simulink model "Demo_TrafficFlow_cs"  (Simulink 7.6)
settings: Solver=ode5  SolverMode=SingleTasking  StartTime=0.0  StopTime=60  FixedStep=1/20  RelTol=1e-3
PreLoadFcn:
  | check_NUTS_defined
  | try
  |     evalin('base', 'NUTS_defined;');
  | catch
  |     warningMsg = sprintf('PreScan Warning: \nUsing this model requires that MATLAB is configured for PreScan. The model may not display correctly.\n\n ==> Please open MATLAB with the dedicated buttons provided with PreScan');
  |     disp(warningMsg)
  |     warndlg(warningMsg,'WindowStyle','Modal');
  | end
  | 
  | model_closed = 0;
  | assignin('base',['model_closed_' bdroot],false);
InitFcn:
  | PreScanExperimentInitFcn(bdroot)
StopFcn:
  | PreScanExperimentStopFcn(bdroot)
undefined symbols (not defined in the file or in its folder): Advised_Speed_L1, Advised_Speed_L2, Advised_Speed_L3, DiscIntNeverNeededParam, spare_10, spare_5, spare_6, spare_7, spare_8, spare_9, State_L2, Throughput_L1, Throughput_L2, Throughput_L3, Velocity_L1, Velocity_L2, Velocity_L3
libraries: simulink x58, PreScanSimulinkLibrary x40, simulink_extras x32, PreScanUsefulBlocks x22, simulink_need_slupdate x3
(part 7 of 20)

== system Demo_TrafficFlow_cs/VISUMatrixSign_HighwayOverhang_2 ==
  MatrixSigns : Inport
  SubMuxVisuMatrixSign : Demux  Outputs=12
  visu_MatrixSign_1 : Subsystem  mask(ToSensorWorld){PortName=MatrixSign_1, PortIndex=690, PortWidth=1, TimeStep=1/20}
      mask prompts: PortName "Port Name [-]"; PortIndex "Index [-]"; PortWidth "Port Dimensions [-]"; TimeStep "Upload Period [s]"
      mask help: Sends signal(s) from Simulink to the Sensor World
      mask Help:
      | Sends signal(s) from Simulink to the Sensor World.
      | 
      | - Port Name [-]: specifies the name of the output terminal of the Simulink block.
      | - Index [-]: specifies the start position in the output vector sent to the Sensor World
      | - Port dimensions [-]: specifies the width (number of scalars) of the output port
      | - Upload period [s]: specifies the upload period (in seconds) of the port.
      mask Display: port_label('input', 1, PortName)
  visu_MatrixSign_10 : Subsystem  mask(ToSensorWorld){PortName=MatrixSign_4, PortIndex=699, PortWidth=1, TimeStep=1/20}
      mask prompts: PortName "Port Name [-]"; PortIndex "Index [-]"; PortWidth "Port Dimensions [-]"; TimeStep "Upload Period [s]"
      mask help: Sends signal(s) from Simulink to the Sensor World
      mask Help:
      | Sends signal(s) from Simulink to the Sensor World.
      | 
      | - Port Name [-]: specifies the name of the output terminal of the Simulink block.
      | - Index [-]: specifies the start position in the output vector sent to the Sensor World
      | - Port dimensions [-]: specifies the width (number of scalars) of the output port
      | - Upload period [s]: specifies the upload period (in seconds) of the port.
      mask Display: port_label('input', 1, PortName)
  visu_MatrixSign_11 : Subsystem  mask(ToSensorWorld){PortName=MatrixSign_5, PortIndex=700, PortWidth=1, TimeStep=1/20}
      mask prompts: PortName "Port Name [-]"; PortIndex "Index [-]"; PortWidth "Port Dimensions [-]"; TimeStep "Upload Period [s]"
      mask help: Sends signal(s) from Simulink to the Sensor World
      mask Help:
      | Sends signal(s) from Simulink to the Sensor World.
      | 
      | - Port Name [-]: specifies the name of the output terminal of the Simulink block.
      | - Index [-]: specifies the start position in the output vector sent to the Sensor World
      | - Port dimensions [-]: specifies the width (number of scalars) of the output port
      | - Upload period [s]: specifies the upload period (in seconds) of the port.
      mask Display: port_label('input', 1, PortName)
  visu_MatrixSign_12 : Subsystem  mask(ToSensorWorld){PortName=MatrixSign_6, PortIndex=701, PortWidth=1, TimeStep=1/20}
      mask prompts: PortName "Port Name [-]"; PortIndex "Index [-]"; PortWidth "Port Dimensions [-]"; TimeStep "Upload Period [s]"
      mask help: Sends signal(s) from Simulink to the Sensor World
      mask Help:
      | Sends signal(s) from Simulink to the Sensor World.
      | 
      | - Port Name [-]: specifies the name of the output terminal of the Simulink block.
      | - Index [-]: specifies the start position in the output vector sent to the Sensor World
      | - Port dimensions [-]: specifies the width (number of scalars) of the output port
      | - Upload period [s]: specifies the upload period (in seconds) of the port.
      mask Display: port_label('input', 1, PortName)
  visu_MatrixSign_2 : Subsystem  mask(ToSensorWorld){PortName=MatrixSign_2, PortIndex=691, PortWidth=1, TimeStep=1/20}
      mask prompts: PortName "Port Name [-]"; PortIndex "Index [-]"; PortWidth "Port Dimensions [-]"; TimeStep "Upload Period [s]"
      mask help: Sends signal(s) from Simulink to the Sensor World
      mask Help:
      | Sends signal(s) from Simulink to the Sensor World.
      | 
      | - Port Name [-]: specifies the name of the output terminal of the Simulink block.
      | - Index [-]: specifies the start position in the output vector sent to the Sensor World
      | - Port dimensions [-]: specifies the width (number of scalars) of the output port
      | - Upload period [s]: specifies the upload period (in seconds) of the port.
      mask Display: port_label('input', 1, PortName)
  visu_MatrixSign_3 : Subsystem  mask(ToSensorWorld){PortName=MatrixSign_3, PortIndex=692, PortWidth=1, TimeStep=1/20}
      mask prompts: PortName "Port Name [-]"; PortIndex "Index [-]"; PortWidth "Port Dimensions [-]"; TimeStep "Upload Period [s]"
      mask help: Sends signal(s) from Simulink to the Sensor World
      mask Help:
      | Sends signal(s) from Simulink to the Sensor World.
      | 
      | - Port Name [-]: specifies the name of the output terminal of the Simulink block.
      | - Index [-]: specifies the start position in the output vector sent to the Sensor World
      | - Port dimensions [-]: specifies the width (number of scalars) of the output port
      | - Upload period [s]: specifies the upload period (in seconds) of the port.
      mask Display: port_label('input', 1, PortName)
  visu_MatrixSign_4 : Subsystem  mask(ToSensorWorld){PortName=MatrixSign_4, PortIndex=693, PortWidth=1, TimeStep=1/20}
      mask prompts: PortName "Port Name [-]"; PortIndex "Index [-]"; PortWidth "Port Dimensions [-]"; TimeStep "Upload Period [s]"
      mask help: Sends signal(s) from Simulink to the Sensor World
      mask Help:
      | Sends signal(s) from Simulink to the Sensor World.
      | 
      | - Port Name [-]: specifies the name of the output terminal of the Simulink block.
      | - Index [-]: specifies the start position in the output vector sent to the Sensor World
      | - Port dimensions [-]: specifies the width (number of scalars) of the output port
      | - Upload period [s]: specifies the upload period (in seconds) of the port.
      mask Display: port_label('input', 1, PortName)
  visu_MatrixSign_5 : Subsystem  mask(ToSensorWorld){PortName=MatrixSign_5, PortIndex=694, PortWidth=1, TimeStep=1/20}
      mask prompts: PortName "Port Name [-]"; PortIndex "Index [-]"; PortWidth "Port Dimensions [-]"; TimeStep "Upload Period [s]"
      mask help: Sends signal(s) from Simulink to the Sensor World
      mask Help:
      | Sends signal(s) from Simulink to the Sensor World.
      | 
      | - Port Name [-]: specifies the name of the output terminal of the Simulink block.
      | - Index [-]: specifies the start position in the output vector sent to the Sensor World
      | - Port dimensions [-]: specifies the width (number of scalars) of the output port
      | - Upload period [s]: specifies the upload period (in seconds) of the port.
      mask Display: port_label('input', 1, PortName)
  visu_MatrixSign_6 : Subsystem  mask(ToSensorWorld){PortName=MatrixSign_6, PortIndex=695, PortWidth=1, TimeStep=1/20}
      mask prompts: PortName "Port Name [-]"; PortIndex "Index [-]"; PortWidth "Port Dimensions [-]"; TimeStep "Upload Period [s]"
      mask help: Sends signal(s) from Simulink to the Sensor World
      mask Help:
      | Sends signal(s) from Simulink to the Sensor World.
      | 
      | - Port Name [-]: specifies the name of the output terminal of the Simulink block.
      | - Index [-]: specifies the start position in the output vector sent to the Sensor World
      | - Port dimensions [-]: specifies the width (number of scalars) of the output port
      | - Upload period [s]: specifies the upload period (in seconds) of the port.
      mask Display: port_label('input', 1, PortName)
  visu_MatrixSign_7 : Subsystem  mask(ToSensorWorld){PortName=MatrixSign_1, PortIndex=696, PortWidth=1, TimeStep=1/20}
      mask prompts: PortName "Port Name [-]"; PortIndex "Index [-]"; PortWidth "Port Dimensions [-]"; TimeStep "Upload Period [s]"
      mask help: Sends signal(s) from Simulink to the Sensor World
      mask Help:
      | Sends signal(s) from Simulink to the Sensor World.
      | 
      | - Port Name [-]: specifies the name of the output terminal of the Simulink block.
      | - Index [-]: specifies the start position in the output vector sent to the Sensor World
      | - Port dimensions [-]: specifies the width (number of scalars) of the output port
      | - Upload period [s]: specifies the upload period (in seconds) of the port.
      mask Display: port_label('input', 1, PortName)
  visu_MatrixSign_8 : Subsystem  mask(ToSensorWorld){PortName=MatrixSign_2, PortIndex=697, PortWidth=1, TimeStep=1/20}
      mask prompts: PortName "Port Name [-]"; PortIndex "Index [-]"; PortWidth "Port Dimensions [-]"; TimeStep "Upload Period [s]"
      mask help: Sends signal(s) from Simulink to the Sensor World
      mask Help:
      | Sends signal(s) from Simulink to the Sensor World.
      | 
      | - Port Name [-]: specifies the name of the output terminal of the Simulink block.
      | - Index [-]: specifies the start position in the output vector sent to the Sensor World
      | - Port dimensions [-]: specifies the width (number of scalars) of the output port
      | - Upload period [s]: specifies the upload period (in seconds) of the port.
      mask Display: port_label('input', 1, PortName)
  visu_MatrixSign_9 : Subsystem  mask(ToSensorWorld){PortName=MatrixSign_3, PortIndex=698, PortWidth=1, TimeStep=1/20}
      mask prompts: PortName "Port Name [-]"; PortIndex "Index [-]"; PortWidth "Port Dimensions [-]"; TimeStep "Upload Period [s]"
      mask help: Sends signal(s) from Simulink to the Sensor World
      mask Help:
      | Sends signal(s) from Simulink to the Sensor World.
      | 
      | - Port Name [-]: specifies the name of the output terminal of the Simulink block.
      | - Index [-]: specifies the start position in the output vector sent to the Sensor World
      | - Port dimensions [-]: specifies the width (number of scalars) of the output port
      | - Upload period [s]: specifies the upload period (in seconds) of the port.
      mask Display: port_label('input', 1, PortName)
  wires:
  SubMuxVisuMatrixSign/1 -> visu_MatrixSign_1/1(Y)
  MatrixSigns/1 -> SubMuxVisuMatrixSign/1
  SubMuxVisuMatrixSign/2 -> visu_MatrixSign_2/1(Y)
  SubMuxVisuMatrixSign/3 -> visu_MatrixSign_3/1(Y)
  SubMuxVisuMatrixSign/4 -> visu_MatrixSign_4/1(Y)
  SubMuxVisuMatrixSign/5 -> visu_MatrixSign_5/1(Y)
  SubMuxVisuMatrixSign/6 -> visu_MatrixSign_6/1(Y)
  SubMuxVisuMatrixSign/7 -> visu_MatrixSign_7/1(Y)
  SubMuxVisuMatrixSign/8 -> visu_MatrixSign_8/1(Y)
  SubMuxVisuMatrixSign/9 -> visu_MatrixSign_9/1(Y)
  SubMuxVisuMatrixSign/10 -> visu_MatrixSign_10/1(Y)
  SubMuxVisuMatrixSign/11 -> visu_MatrixSign_11/1(Y)
  SubMuxVisuMatrixSign/12 -> visu_MatrixSign_12/1(Y)

== system Demo_TrafficFlow_cs/Audi_A8_1/AIR_1_Demux ==
  AIR : Inport
  Activate_Switch : Subsystem
      mask Display: text(5,10,'')
  Bus Selector : BusSelector  OutputSignals=DATA.Range,DATA.Azimuth,DATA.Elevation,DATA.ID,DATA.Velocity,DATA.Heading
  Data Type Conversion : DataTypeConversion  OutDataTypeStr=double
  For Iterator : ForIterator  ResetStates=reset  IterationLimit=detectableObjects
  Selector : Selector  InputPortWidth=detectableObjects  IndexOptions=Index vector (port)  Indices=[1 3]  OutputSizes=1
  Signal2ArrayOfSignals1 : Subsystem  mask{VECTORLENGTH=detectableObjects}
      mask prompts: VECTORLENGTH "Length of vector"
  Signal2ArrayOfSignals2 : Subsystem  mask{VECTORLENGTH=detectableObjects}
      mask prompts: VECTORLENGTH "Length of vector"
  Signal2ArrayOfSignals3 : Subsystem  mask{VECTORLENGTH=detectableObjects}
      mask prompts: VECTORLENGTH "Length of vector"
  Signal2ArrayOfSignals4 : Subsystem  mask{VECTORLENGTH=detectableObjects}
      mask prompts: VECTORLENGTH "Length of vector"
  Signal2ArrayOfSignals5 : Subsystem  mask{VECTORLENGTH=detectableObjects}
      mask prompts: VECTORLENGTH "Length of vector"
  Signal2ArrayOfSignals6 : Subsystem  mask{VECTORLENGTH=detectableObjects}
      mask prompts: VECTORLENGTH "Length of vector"
  Range [m] : Outport
  Azimuth [deg] : Outport  Port=2
  Elevation [deg] : Outport  Port=3
  ID [-] : Outport  Port=4
  Velocity [m/s] : Outport  Port=5
  Heading [deg] : Outport  Port=6
  wires:
  Data Type Conversion/1 -> ID [-]/1
  Bus Selector/6 -> Signal2ArrayOfSignals6/2(Element)  "<Heading>"
  Signal2ArrayOfSignals6/1(ArrayofElements) -> Activate_Switch/2(Heading)
  Bus Selector/5 -> Signal2ArrayOfSignals5/2(Element)  "<Velocity>"
  Signal2ArrayOfSignals5/1(ArrayofElements) -> Velocity [m/s]/1
  Bus Selector/4 -> Signal2ArrayOfSignals4/2(Element)  "<ID>"
  Signal2ArrayOfSignals4/1(ArrayofElements) -> Data Type Conversion/1, Activate_Switch/1(BeamID)
  Bus Selector/3 -> Signal2ArrayOfSignals3/2(Element)  "<Elevation>"
  Signal2ArrayOfSignals3/1(ArrayofElements) -> Elevation [deg]/1
  Bus Selector/2 -> Signal2ArrayOfSignals1/2(Element)  "<Azimuth>"
  Signal2ArrayOfSignals1/1(ArrayofElements) -> Azimuth [deg]/1
  Signal2ArrayOfSignals2/1(ArrayofElements) -> Range [m]/1
  Bus Selector/1 -> Signal2ArrayOfSignals2/2(Element)  "<Range>"
  Selector/1 -> Bus Selector/1
  For Iterator/1 -> Signal2ArrayOfSignals6/1(LoopIndex), Signal2ArrayOfSignals5/1(LoopIndex), Signal2ArrayOfSignals4/1(LoopIndex), Signal2ArrayOfSignals3/1(LoopIndex), Signal2ArrayOfSignals1/1(LoopIndex), Signal2ArrayOfSignals2/1(LoopIndex), Selector/2
  AIR/1 -> Selector/1
  Activate_Switch/1(On/Off) -> Heading [deg]/1

== system Demo_TrafficFlow_cs/Audi_A8_1/RFOBU_1_Rx_Demux ==
  Beacon_Rx : Inport
  Demux1 : Demux  Outputs=3
  Demux2 : Demux  Outputs=20
  Mux : Mux  Inputs=3
  Reorder : Subsystem  mask(Output reorder block){NrOfOutputs=20, NrOfObjects=2}
      mask prompts: NrOfOutputs "Number Of Outputs [-]"; NrOfObjects "Number Of Objects [-]"
      mask help: Change output order from object based to output type based
      mask init:
      | InputPortWidth=NrOfObjects*NrOfOutputs;
      | Input=1:InputPortWidth;
      | ReshapedInput=reshape(Input,NrOfOutputs,NrOfObjects);
      | TransposedReshapedInput=transpose(ReshapedInput);
      | Elements=reshape(TransposedReshapedInput,length(Input),1);
  xyz2GPS : PreScanUsefulBlocks/xyz2GPS  CoordinateCount=2  GPS_base=[0.000000 0.000000 0.000]
  Beacon_ID : Outport
  GPS_pos_lat_[deg] : Outport  Port=2
  GPS_pos_long_[deg] : Outport  Port=3
  GPS_pos_alt_[m] : Outport  Port=4
  BeaconType [-] : Outport  Port=5
  spare_1 : Outport  Port=6
  spare_2 : Outport  Port=7
  spare_3 : Outport  Port=8
  spare_4 : Outport  Port=9
  spare_5 : Outport  Port=10
  spare_6 : Outport  Port=11
  spare_7 : Outport  Port=12
  spare_8 : Outport  Port=13
  spare_9 : Outport  Port=14
  spare_10 : Outport  Port=15
  spare_11 : Outport  Port=16
  spare_12 : Outport  Port=17
  spare_13 : Outport  Port=18
  spare_14 : Outport  Port=19
  spare_15 : Outport  Port=20
  wires:
  Demux2/5 -> BeaconType [-]/1  "pos_z"
  Mux/1 -> xyz2GPS/1
  Demux2/20 -> spare_15/1
  Demux2/19 -> spare_14/1
  Demux2/18 -> spare_13/1
  Demux2/17 -> spare_12/1
  Demux2/16 -> spare_11/1
  Beacon_Rx/1 -> Reorder/1(In)
  Demux2/15 -> spare_10/1
  Demux2/14 -> spare_9/1
  Demux2/13 -> spare_8/1
  Demux2/12 -> spare_7/1
  Demux2/11 -> spare_6/1
  Demux2/10 -> spare_5/1
  Demux2/9 -> spare_4/1
  Demux2/8 -> spare_3/1
  Demux2/7 -> spare_2/1
  Demux2/6 -> spare_1/1  "pos_z"
  Reorder/1(Out) -> Demux2/1
  Demux1/3 -> GPS_pos_alt_[m]/1
  Demux1/2 -> GPS_pos_long_[deg]/1
  Demux1/1 -> GPS_pos_lat_[deg]/1
  xyz2GPS/1 -> Demux1/1
  Demux2/4 -> Mux/3  "pos_z"
  Demux2/3 -> Mux/2  "pos_y"
  Demux2/2 -> Mux/1  "pos_x"
  Demux2/1 -> Beacon_ID/1

== system Demo_TrafficFlow_cs/Audi_A8_1/RFOBU_1_Tx_Mux ==
  Vehicle_Type : Inport
  spare_1 : Inport  Port=2
  spare_2 : Inport  Port=3
  spare_3 : Inport  Port=4
  spare_4 : Inport  Port=5
  spare_5 : Inport  Port=6
  spare_6 : Inport  Port=7
  spare_7 : Inport  Port=8
  spare_8 : Inport  Port=9
  spare_9 : Inport  Port=10
  spare_10 : Inport  Port=11
  spare_11 : Inport  Port=12
  spare_12 : Inport  Port=13
  spare_13 : Inport  Port=14
  spare_14 : Inport  Port=15
  spare_15 : Inport  Port=16
  Mux1 : Mux  Inputs=16
  BeaconTx : Outport
  wires:
  Vehicle_Type/1 -> Mux1/1
  Mux1/1 -> BeaconTx/1
  spare_10/1 -> Mux1/11
  spare_9/1 -> Mux1/10
  spare_8/1 -> Mux1/9
  spare_7/1 -> Mux1/8
  spare_6/1 -> Mux1/7
  spare_5/1 -> Mux1/6
  spare_4/1 -> Mux1/5
  spare_3/1 -> Mux1/4
  spare_2/1 -> Mux1/3
  spare_1/1 -> Mux1/2
  spare_15/1 -> Mux1/16
  spare_14/1 -> Mux1/15
  spare_13/1 -> Mux1/14
  spare_12/1 -> Mux1/13
  spare_11/1 -> Mux1/12

== system Demo_TrafficFlow_cs/Audi_A8_1/SELF_Demux ==
  SELF : Inport
  Demux1 : Demux  Outputs=3
  Demux2 : Demux  Outputs=3
  Demux3 : Demux  Outputs=[3 3 1 1]
  Demux4 : Demux  Outputs=3
  Gain : Gain  Gain=180/pi
  Gain1 : Gain  Gain=180/pi
  Selector : Selector  InputPortWidth=3  IndexOptions=Index vector (dialog)  Indices=[3]  OutputSizes=1
  compas converter : Subsystem
  xyz2GPS : PreScanUsefulBlocks/xyz2GPS  CoordinateCount=1  GPS_base=[0.000000 0.000000 0.000]
  x [m] : Outport
  y [m] : Outport  Port=2
  z [m] : Outport  Port=3
  rotx [deg] : Outport  Port=4
  roty [deg] : Outport  Port=5
  rotz [deg] : Outport  Port=6
  GPS lat [deg] : Outport  Port=7
  GPS long [deg] : Outport  Port=8
  GPS alt [m] : Outport  Port=9
  velocity [m/s] : Outport  Port=10
  heading [deg] : Outport  Port=11
  yaw rate [deg/s] : Outport  Port=12
  wires:
  Demux3/4 -> Gain1/1
  Gain1/1 -> yaw rate [deg/s]/1
  Demux3/3 -> velocity [m/s]/1
  compas converter/1(heading [deg]) -> heading [deg]/1
  xyz2GPS/1 -> Demux4/1
  Demux4/3 -> GPS alt [m]/1
  Demux4/2 -> GPS long [deg]/1
  Demux4/1 -> GPS lat [deg]/1
  Demux3/2 -> Gain/1, Selector/1
  Demux3/1 -> xyz2GPS/1, Demux1/1
  SELF/1 -> Demux3/1
  Demux2/3 -> rotz [deg]/1
  Demux2/2 -> roty [deg]/1
  Demux2/1 -> rotx [deg]/1
  Demux1/3 -> z [m]/1
  Demux1/2 -> y [m]/1
  Demux1/1 -> x [m]/1
  Gain/1 -> Demux2/1
  Selector/1 -> compas converter/1(rotz [rad])

== system Demo_TrafficFlow_cs/Audi_A8_1/Simple_Longitudinal_ACC ==
  note: v_ini=50 km/h
  Advised Velocity [km/h] : Inport
  Range [m] : Inport  Port=2
  Velocity [m/s] : Inport  Port=3
  HOLD Advised Speed : Subsystem
  HardCoded Safety Distance [m] : Constant  Value=15
  LONGITUDINAL ACC & Dynamics : Subsystem
  Manual Switch : simulink/Signal Routing/Manual Switch  SystemSampleTime=-1  sw=1  action=0  varsize=off
  Manual Switch1 : simulink/Signal Routing/Manual Switch  SystemSampleTime=-1  sw=1  action=0  varsize=off
  Memory2 : Memory
  Own Speed Intention [km/h] : Constant  Value=60
  Product1 : Product  RndMeth=Zero
  TimeToCollision [s] Velocity Dependent Safety Distance : Constant
  ACC_Velocity [m/s] : Outport
  wires:
  Range [m]/1 -> LONGITUDINAL ACC & Dynamics/1(Detected Range [m])
  TimeToCollision [s] Velocity Dependent Safety Distance/1 -> Product1/1
  HardCoded Safety Distance [m]/1 -> Manual Switch/1
  Product1/1 -> Manual Switch/2
  Manual Switch/1 -> LONGITUDINAL ACC & Dynamics/4(Safety Distance [m])
  Advised Velocity [km/h]/1 -> HOLD Advised Speed/1(v)
  Own Speed Intention [km/h]/1 -> Manual Switch1/2
  HOLD Advised Speed/1(v_hold) -> Manual Switch1/1
  Manual Switch1/1 -> LONGITUDINAL ACC & Dynamics/3(Own Velocity Intention [km/h])
  LONGITUDINAL ACC & Dynamics/1(Velocity [m/s]) -> ACC_Velocity [m/s]/1, Memory2/1
  Velocity [m/s]/1 -> LONGITUDINAL ACC & Dynamics/2(Detected Velocity Vehicle [m/s])
  Memory2/1 -> Product1/2

== system Demo_TrafficFlow_cs/Audi_A8_1/Trajectories ==
  Velocity : Inport
  OBUID_Reinsert [-] : Inport  Port=2
  StateLANE2 [-] : Inport  Port=3
  END OF TRACK REACHED & GO TO BUFFER : Subsystem
  ElapsedTrack1 : Subsystem  Tag=ElapsedTrack  mask(PreScan Demux Block:){Filename='PathFollowerSva_2_Path.mat'}
      mask prompts: Filename "Name path-file"
      mask init:
      | route=load(['trajectories/',Filename]);
      | 
      | s=route.path(1,:);
      | x=route.path(2,:);
      | y=route.path(3,:);
      | z=route.path(4,:);
      | roll=route.path(5,:);
      | pitch=route.path(6,:);
      | yaw=route.path(7,:);
      settings: DeleteFcn=PreScanBlockDeleteFcn;
  Integrator : Integrator  ExternalReset=level  InitialConditionSource=external  LimitOutput=on  UpperSaturationLimit=180  LowerSaturationLimit=-1e-3
  Integrator1 : DiscreteIntegrator  Tag=TrajIntegrator  SampleTime=1/20  ICPrevOutput=DiscIntNeverNeededParam  ICPrevScaledInput=DiscIntNeverNeededParam
  LANE CHANGE LEFT (cummulative y position;  multiple shift to left are possible) : Subsystem  mask{Filename='Path_LC_Left.mat'}
      mask prompts: Filename "Name path-file"
      mask init:
      | %route=load(['Trajectories/',Filename]);
      | route=load(Filename);
      | s=route.path(1,:);
      | x=route.path(2,:);
      | y=route.path(3,:);
      | yaw=route.path(4,:);
  Lane 1 : Constant
  Length_of_Track [m] : Constant  Value=180
  OBU_ID : Constant  Value=10
  PathFollowerSva_2 : Subsystem  Tag=Trajectory  mask(PreScan Source Block: Trajectory){FileName='PathFollowerSva_2.mat', TimeStep=1/20}
      mask prompts: TimeStep "Time Step [s]"
      mask help:
      | The Trajectory block contains the trajectories defined in the PreScan Experiment Editor. The output of the trajectory block is the (vehicle) State.
      |
      | For more information, please click Help.
      mask init:
      | [direc,name]=fileparts(get_param(bdroot, 'FileName'));
      | load([direc,'/Trajectories/',FileName])
      | trajectory=trajectory';
      | 
      | traj.time=trajectory(:,1);%[];
      | traj.signals.values=trajectory(:,2:19);
      | traj.dimensions=18;
      | traj.label='';
      | 
      | SampleTime=traj.time(2)-traj.time(1);
      settings: DeleteFcn=PreScanBlockDeleteFcn;
      mask Help: eval('openHelp(312)')
      mask Display: fprintf(FileName)
  PathFollowerSva_2_Offset : Constant  Tag=TrajectoryOffset  Value="[0  0  0  0  0  0]"
  Product : Product  RndMeth=Zero
  Product1 : Product  RndMeth=Zero
  RESTART TRAJECT : Subsystem
  Random  LC generator : Subsystem
  Relational Operator2 : RelationalOperator  Operator===  OutDataTypeStr=boolean
  Selector1 : Constant  Tag=TrajSubSelector  Value=2
  Step : Step  Time=0.01  Before=1  After=0  SampleTime=0
  Sum : Sum  Inputs=|+-
  Sum2 : Sum  Inputs=|++
  Terminator_1 : Terminator
  Traj2State1 : Subsystem  Tag=Traj2State
      settings: DeleteFcn=PreScanBlockDeleteFcn;
      mask Type: PreScan Demux Block:
  TrajSwitch1 : MultiPortSwitch  Tag=TrajSubSwitch  Inputs=2  zeroidx=off
  Velocity1 : Constant  Tag=TrajVelocity  Value=15
  x0 : Constant  Value=0
  State : Outport  Tag=TrajOutport
  wires:
  END OF TRACK REACHED & GO TO BUFFER/1(Buffer_offset [m]) -> Sum/2
  LANE CHANGE LEFT (cummulative y position;  multiple shift to left are possible)/1(cum LC) -> Sum2/2  "Add Lane Change"
  Sum/1 -> Sum2/1
  RESTART TRAJECT/1(reset [-]) -> [reset]
  END OF TRACK REACHED & GO TO BUFFER/2(End_of_Track_Reached [-]) -> RESTART TRAJECT/1(End_of_Track_Reached [-])
  Step/1 -> Product/1
  x0/1 -> Product/2
  OBUID_Reinsert [-]/1 -> RESTART TRAJECT/2(ID_start_again [-])
  OBU_ID/1 -> RESTART TRAJECT/3(objectID [-])
  Lane 1/1 -> Relational Operator2/2
  [reset] -> LANE CHANGE LEFT (cummulative y position;  multiple shift to left are possible)/1(reset cum value)
  Relational Operator2/1 -> LANE CHANGE LEFT (cummulative y position;  multiple shift to left are possible)/3(on/off [-])
  Length_of_Track [m]/1 -> END OF TRACK REACHED & GO TO BUFFER/2(Length_of_Track [m])
  Random  LC generator/1(0/1) -> Product1/2
  StateLANE2 [-]/1 -> Product1/1
  Product1/1 -> Relational Operator2/1
  RESTART TRAJECT/2(Start_Traject [-]) -> Integrator/2
  Product/1 -> Integrator/3
  Velocity/1 -> Integrator/1, LANE CHANGE LEFT (cummulative y position;  multiple shift to left are possible)/2(velocity [m/s])
  Integrator/1 -> END OF TRACK REACHED & GO TO BUFFER/1(Elapsed_Track [m]), ElapsedTrack1/2(s)
  PathFollowerSva_2/1(trajectory) -> Traj2State1/1(Traj Org)
  PathFollowerSva_2_Offset/1 -> Traj2State1/2(Offset), ElapsedTrack1/1(Offset)
  Velocity1/1 -> Integrator1/1
  Selector1/1 -> TrajSwitch1/1
  Traj2State1/1(state) -> TrajSwitch1/2
  ElapsedTrack1/1(state) -> Sum/1
  TrajSwitch1/1 -> State/1
  Sum2/1 -> TrajSwitch1/3
  Integrator1/1 -> Terminator_1/1

== system Demo_TrafficFlow_cs/BMW_X5_1/AIR_2_Demux ==
  AIR : Inport
  Activate_Switch : Subsystem
      mask Display: text(5,10,'')
  Bus Selector : BusSelector  OutputSignals=DATA.Range,DATA.Azimuth,DATA.Elevation,DATA.ID,DATA.Velocity,DATA.Heading
  Data Type Conversion : DataTypeConversion  OutDataTypeStr=double
  For Iterator : ForIterator  ResetStates=reset  IterationLimit=detectableObjects
  Selector : Selector  InputPortWidth=detectableObjects  IndexOptions=Index vector (port)  Indices=[1 3]  OutputSizes=1
  Signal2ArrayOfSignals1 : Subsystem  mask{VECTORLENGTH=detectableObjects}
      mask prompts: VECTORLENGTH "Length of vector"
  Signal2ArrayOfSignals2 : Subsystem  mask{VECTORLENGTH=detectableObjects}
      mask prompts: VECTORLENGTH "Length of vector"
  Signal2ArrayOfSignals3 : Subsystem  mask{VECTORLENGTH=detectableObjects}
      mask prompts: VECTORLENGTH "Length of vector"
  Signal2ArrayOfSignals4 : Subsystem  mask{VECTORLENGTH=detectableObjects}
      mask prompts: VECTORLENGTH "Length of vector"
  Signal2ArrayOfSignals5 : Subsystem  mask{VECTORLENGTH=detectableObjects}
      mask prompts: VECTORLENGTH "Length of vector"
  Signal2ArrayOfSignals6 : Subsystem  mask{VECTORLENGTH=detectableObjects}
      mask prompts: VECTORLENGTH "Length of vector"
  Range [m] : Outport
  Azimuth [deg] : Outport  Port=2
  Elevation [deg] : Outport  Port=3
  ID [-] : Outport  Port=4
  Velocity [m/s] : Outport  Port=5
  Heading [deg] : Outport  Port=6
  wires:
  Data Type Conversion/1 -> ID [-]/1
  Bus Selector/6 -> Signal2ArrayOfSignals6/2(Element)  "<Heading>"
  Signal2ArrayOfSignals6/1(ArrayofElements) -> Activate_Switch/2(Heading)
  Bus Selector/5 -> Signal2ArrayOfSignals5/2(Element)  "<Velocity>"
  Signal2ArrayOfSignals5/1(ArrayofElements) -> Velocity [m/s]/1
  Bus Selector/4 -> Signal2ArrayOfSignals4/2(Element)  "<ID>"
  Signal2ArrayOfSignals4/1(ArrayofElements) -> Data Type Conversion/1, Activate_Switch/1(BeamID)
  Bus Selector/3 -> Signal2ArrayOfSignals3/2(Element)  "<Elevation>"
  Signal2ArrayOfSignals3/1(ArrayofElements) -> Elevation [deg]/1
  Bus Selector/2 -> Signal2ArrayOfSignals1/2(Element)  "<Azimuth>"
  Signal2ArrayOfSignals1/1(ArrayofElements) -> Azimuth [deg]/1
  Signal2ArrayOfSignals2/1(ArrayofElements) -> Range [m]/1
  Bus Selector/1 -> Signal2ArrayOfSignals2/2(Element)  "<Range>"
  Selector/1 -> Bus Selector/1
  For Iterator/1 -> Signal2ArrayOfSignals6/1(LoopIndex), Signal2ArrayOfSignals5/1(LoopIndex), Signal2ArrayOfSignals4/1(LoopIndex), Signal2ArrayOfSignals3/1(LoopIndex), Signal2ArrayOfSignals1/1(LoopIndex), Signal2ArrayOfSignals2/1(LoopIndex), Selector/2
  AIR/1 -> Selector/1
  Activate_Switch/1(On/Off) -> Heading [deg]/1

== system Demo_TrafficFlow_cs/BMW_X5_1/RFOBU_2_Rx_Demux ==
  Beacon_Rx : Inport
  Demux1 : Demux  Outputs=3
  Demux2 : Demux  Outputs=20
  Mux : Mux  Inputs=3
  Reorder : Subsystem  mask(Output reorder block){NrOfOutputs=20, NrOfObjects=2}
      mask prompts: NrOfOutputs "Number Of Outputs [-]"; NrOfObjects "Number Of Objects [-]"
      mask help: Change output order from object based to output type based
      mask init: same as Demo_TrafficFlow_cs/Audi_A8_1/RFOBU_1_Rx_Demux/Reorder
  xyz2GPS : PreScanUsefulBlocks/xyz2GPS  CoordinateCount=2  GPS_base=[0.000000 0.000000 0.000]
  Beacon_ID : Outport
  GPS_pos_lat_[deg] : Outport  Port=2
  GPS_pos_long_[deg] : Outport  Port=3
  GPS_pos_alt_[m] : Outport  Port=4
  BeaconType [-] : Outport  Port=5
  spare_1 : Outport  Port=6
  spare_2 : Outport  Port=7
  spare_3 : Outport  Port=8
  spare_4 : Outport  Port=9
  spare_5 : Outport  Port=10
  spare_6 : Outport  Port=11
  spare_7 : Outport  Port=12
  spare_8 : Outport  Port=13
  spare_9 : Outport  Port=14
  spare_10 : Outport  Port=15
  spare_11 : Outport  Port=16
  spare_12 : Outport  Port=17
  spare_13 : Outport  Port=18
  spare_14 : Outport  Port=19
  spare_15 : Outport  Port=20
  wires:
  Demux2/5 -> BeaconType [-]/1  "pos_z"
  Mux/1 -> xyz2GPS/1
  Demux2/20 -> spare_15/1
  Demux2/19 -> spare_14/1
  Demux2/18 -> spare_13/1
  Demux2/17 -> spare_12/1
  Demux2/16 -> spare_11/1
  Beacon_Rx/1 -> Reorder/1(In)
  Demux2/15 -> spare_10/1
  Demux2/14 -> spare_9/1
  Demux2/13 -> spare_8/1
  Demux2/12 -> spare_7/1
  Demux2/11 -> spare_6/1
  Demux2/10 -> spare_5/1
  Demux2/9 -> spare_4/1
  Demux2/8 -> spare_3/1
  Demux2/7 -> spare_2/1
  Demux2/6 -> spare_1/1  "pos_z"
  Reorder/1(Out) -> Demux2/1
  Demux1/3 -> GPS_pos_alt_[m]/1
  Demux1/2 -> GPS_pos_long_[deg]/1
  Demux1/1 -> GPS_pos_lat_[deg]/1
  xyz2GPS/1 -> Demux1/1
  Demux2/4 -> Mux/3  "pos_z"
  Demux2/3 -> Mux/2  "pos_y"
  Demux2/2 -> Mux/1  "pos_x"
  Demux2/1 -> Beacon_ID/1

== system Demo_TrafficFlow_cs/BMW_X5_1/RFOBU_2_Tx_Mux ==
  Vehicle_Type : Inport
  spare_1 : Inport  Port=2
  spare_2 : Inport  Port=3
  spare_3 : Inport  Port=4
  spare_4 : Inport  Port=5
  spare_5 : Inport  Port=6
  spare_6 : Inport  Port=7
  spare_7 : Inport  Port=8
  spare_8 : Inport  Port=9
  spare_9 : Inport  Port=10
  spare_10 : Inport  Port=11
  spare_11 : Inport  Port=12
  spare_12 : Inport  Port=13
  spare_13 : Inport  Port=14
  spare_14 : Inport  Port=15
  spare_15 : Inport  Port=16
  Mux1 : Mux  Inputs=16
  BeaconTx : Outport
  wires:
  Vehicle_Type/1 -> Mux1/1
  Mux1/1 -> BeaconTx/1
  spare_10/1 -> Mux1/11
  spare_9/1 -> Mux1/10
  spare_8/1 -> Mux1/9
  spare_7/1 -> Mux1/8
  spare_6/1 -> Mux1/7
  spare_5/1 -> Mux1/6
  spare_4/1 -> Mux1/5
  spare_3/1 -> Mux1/4
  spare_2/1 -> Mux1/3
  spare_1/1 -> Mux1/2
  spare_15/1 -> Mux1/16
  spare_14/1 -> Mux1/15
  spare_13/1 -> Mux1/14
  spare_12/1 -> Mux1/13
  spare_11/1 -> Mux1/12

== system Demo_TrafficFlow_cs/BMW_X5_1/SELF_Demux ==
  SELF : Inport
  Demux1 : Demux  Outputs=3
  Demux2 : Demux  Outputs=3
  Demux3 : Demux  Outputs=[3 3 1 1]
  Demux4 : Demux  Outputs=3
  Gain : Gain  Gain=180/pi
  Gain1 : Gain  Gain=180/pi
  Selector : Selector  InputPortWidth=3  IndexOptions=Index vector (dialog)  Indices=[3]  OutputSizes=1
  compas converter : Subsystem
  xyz2GPS : PreScanUsefulBlocks/xyz2GPS  CoordinateCount=1  GPS_base=[0.000000 0.000000 0.000]
  x [m] : Outport
  y [m] : Outport  Port=2
  z [m] : Outport  Port=3
  rotx [deg] : Outport  Port=4
  roty [deg] : Outport  Port=5
  rotz [deg] : Outport  Port=6
  GPS lat [deg] : Outport  Port=7
  GPS long [deg] : Outport  Port=8
  GPS alt [m] : Outport  Port=9
  velocity [m/s] : Outport  Port=10
  heading [deg] : Outport  Port=11
  yaw rate [deg/s] : Outport  Port=12
  wires:
  Demux3/4 -> Gain1/1
  Gain1/1 -> yaw rate [deg/s]/1
  Demux3/3 -> velocity [m/s]/1
  compas converter/1(heading [deg]) -> heading [deg]/1
  xyz2GPS/1 -> Demux4/1
  Demux4/3 -> GPS alt [m]/1
  Demux4/2 -> GPS long [deg]/1
  Demux4/1 -> GPS lat [deg]/1
  Demux3/2 -> Gain/1, Selector/1
  Demux3/1 -> xyz2GPS/1, Demux1/1
  SELF/1 -> Demux3/1
  Demux2/3 -> rotz [deg]/1
  Demux2/2 -> roty [deg]/1
  Demux2/1 -> rotx [deg]/1
  Demux1/3 -> z [m]/1
  Demux1/2 -> y [m]/1
  Demux1/1 -> x [m]/1
  Gain/1 -> Demux2/1
  Selector/1 -> compas converter/1(rotz [rad])

== system Demo_TrafficFlow_cs/BMW_X5_1/Simple_Longitudinal_ACC ==
  note: v_ini=50 km/h
  Advised Velocity [km/h] : Inport
  Range [m] : Inport  Port=2
  Velocity [m/s] : Inport  Port=3
  HOLD Advised Speed : Subsystem
  HardCoded Safety Distance [m] : Constant  Value=15
  LONGITUDINAL ACC & Dynamics : Subsystem
  Manual Switch : simulink/Signal Routing/Manual Switch  SystemSampleTime=-1  sw=1  action=0  varsize=off
  Manual Switch1 : simulink/Signal Routing/Manual Switch  SystemSampleTime=-1  sw=0  action=0  varsize=off
  Memory2 : Memory
  Own Speed Intention [km/h] : Constant  Value=100
  Product1 : Product  RndMeth=Zero
  TimeToCollision [s] Velocity Dependent Safety Distance : Constant
  ACC_Velocity [m/s] : Outport
  wires:
  Range [m]/1 -> LONGITUDINAL ACC & Dynamics/1(Detected Range [m])
  TimeToCollision [s] Velocity Dependent Safety Distance/1 -> Product1/1
  HardCoded Safety Distance [m]/1 -> Manual Switch/1
  Product1/1 -> Manual Switch/2
  Manual Switch/1 -> LONGITUDINAL ACC & Dynamics/4(Safety Distance [m])
  Advised Velocity [km/h]/1 -> HOLD Advised Speed/1(v)
  Own Speed Intention [km/h]/1 -> Manual Switch1/2
  HOLD Advised Speed/1(v_hold) -> Manual Switch1/1
  Manual Switch1/1 -> LONGITUDINAL ACC & Dynamics/3(Own Velocity Intention [km/h])
  LONGITUDINAL ACC & Dynamics/1(Velocity [m/s]) -> ACC_Velocity [m/s]/1, Memory2/1
  Velocity [m/s]/1 -> LONGITUDINAL ACC & Dynamics/2(Detected Velocity Vehicle [m/s])
  Memory2/1 -> Product1/2

== system Demo_TrafficFlow_cs/BMW_X5_1/Trajectories ==
  Velocity : Inport
  OBUID_Reinsert [-] : Inport  Port=2
  StateLANE2 [-] : Inport  Port=3
  END OF TRACK REACHED & GO TO BUFFER : Subsystem
  ElapsedTrack1 : Subsystem  Tag=ElapsedTrack  mask(PreScan Demux Block:){Filename='PathFollowerSva_1_Path.mat'}
      mask prompts: Filename "Name path-file"
      mask init:
      | route=load(['trajectories/',Filename]);
      | 
      | s=route.path(1,:);
      | x=route.path(2,:);
      | y=route.path(3,:);
      | z=route.path(4,:);
      | roll=route.path(5,:);
      | pitch=route.path(6,:);
      | yaw=route.path(7,:);
      settings: DeleteFcn=PreScanBlockDeleteFcn;
  Integrator : Integrator  ExternalReset=level  InitialConditionSource=external  LimitOutput=on  UpperSaturationLimit=180  LowerSaturationLimit=-1e-3
  Integrator1 : DiscreteIntegrator  Tag=TrajIntegrator  SampleTime=1/20  ICPrevOutput=DiscIntNeverNeededParam  ICPrevScaledInput=DiscIntNeverNeededParam
  LANE CHANGE LEFT (cummulative y position;  multiple shift to right are possible) : Subsystem  mask{Filename='Path_LC_Left.mat'}
      mask prompts: Filename "Name path-file"
      mask init:
      | %route=load(['Trajectories/',Filename]);
      | route=load(Filename);
      | s=route.path(1,:);
      | x=route.path(2,:);
      | y=route.path(3,:);
      | yaw=route.path(4,:);
  Lane 3 : Constant  Value=3
  Length_of_Track [m] : Constant  Value=180
  OBU_ID : Constant  Value=11
  PathFollowerSva_1 : Subsystem  Tag=Trajectory  mask(PreScan Source Block: Trajectory){FileName='PathFollowerSva_1.mat', TimeStep=1/20}
      mask prompts: TimeStep "Time Step [s]"
      mask help:
      | The Trajectory block contains the trajectories defined in the PreScan Experiment Editor. The output of the trajectory block is the (vehicle) State.
      |
      | For more information, please click Help.
      mask init: same as Demo_TrafficFlow_cs/Audi_A8_1/Trajectories/PathFollowerSva_2
      settings: DeleteFcn=PreScanBlockDeleteFcn;
      mask Help: eval('openHelp(312)')
      mask Display: fprintf(FileName)
  PathFollowerSva_1_Offset : Constant  Tag=TrajectoryOffset  Value="[0  0  0  0  0  0]"
  Product : Product  RndMeth=Zero
  Product1 : Product  RndMeth=Zero
  RESTART TRAJECT : Subsystem
  Random  LC generator : Subsystem
  Relational Operator2 : RelationalOperator  Operator===  OutDataTypeStr=boolean
  Selector1 : Constant  Tag=TrajSubSelector  Value=2
  Step : Step  Time=0.01  Before=1  After=0  SampleTime=0
  Sum : Sum  Inputs=|+-
  Sum2 : Sum  Inputs=|++
  Terminator_1 : Terminator
  Traj2State1 : Subsystem  Tag=Traj2State
      settings: DeleteFcn=PreScanBlockDeleteFcn;
      mask Type: PreScan Demux Block:
  TrajSwitch1 : MultiPortSwitch  Tag=TrajSubSwitch  Inputs=2  zeroidx=off
  Velocity1 : Constant  Tag=TrajVelocity  Value=15
  x0 : Constant  Value=0
  State : Outport  Tag=TrajOutport
  wires:
  END OF TRACK REACHED & GO TO BUFFER/1(Buffer_offset [m]) -> Sum/2
  LANE CHANGE LEFT (cummulative y position;  multiple shift to right are possible)/1(cum LC) -> Sum2/2  "Add Lane Change"
  RESTART TRAJECT/1(reset [-]) -> [reset]
  END OF TRACK REACHED & GO TO BUFFER/2(End_of_Track_Reached [-]) -> RESTART TRAJECT/1(End_of_Track_Reached [-])
  Step/1 -> Product/1
  x0/1 -> Product/2
  OBUID_Reinsert [-]/1 -> RESTART TRAJECT/2(ID_start_again [-])
  OBU_ID/1 -> RESTART TRAJECT/3(objectID [-])
  Lane 3/1 -> Relational Operator2/2
  [reset] -> LANE CHANGE LEFT (cummulative y position;  multiple shift to right are possible)/1(reset cum value)
  Relational Operator2/1 -> LANE CHANGE LEFT (cummulative y position;  multiple shift to right are possible)/3(on/off [-])
  Length_of_Track [m]/1 -> END OF TRACK REACHED & GO TO BUFFER/2(Length_of_Track [m])
  Sum/1 -> Sum2/1
  Random  LC generator/1(0/1) -> Product1/2
  StateLANE2 [-]/1 -> Product1/1
  Product1/1 -> Relational Operator2/1
  RESTART TRAJECT/2(Start_Traject [-]) -> Integrator/2
  Product/1 -> Integrator/3
  Velocity/1 -> Integrator/1, LANE CHANGE LEFT (cummulative y position;  multiple shift to right are possible)/2(velocity [m/s])
  Integrator/1 -> END OF TRACK REACHED & GO TO BUFFER/1(Elapsed_Track [m]), ElapsedTrack1/2(s)
  PathFollowerSva_1/1(trajectory) -> Traj2State1/1(Traj Org)
  PathFollowerSva_1_Offset/1 -> Traj2State1/2(Offset), ElapsedTrack1/1(Offset)
  Velocity1/1 -> Integrator1/1
  Selector1/1 -> TrajSwitch1/1
  Traj2State1/1(state) -> TrajSwitch1/2
  ElapsedTrack1/1(state) -> Sum/1
  TrajSwitch1/1 -> State/1
  Sum2/1 -> TrajSwitch1/3
  Integrator1/1 -> Terminator_1/1

== system Demo_TrafficFlow_cs/Citroen_C3_1/AIR_3_Demux ==
  AIR : Inport
  Activate_Switch : Subsystem
      mask Display: text(5,10,'')
  Bus Selector : BusSelector  OutputSignals=DATA.Range,DATA.Azimuth,DATA.Elevation,DATA.ID,DATA.Velocity,DATA.Heading
  Data Type Conversion : DataTypeConversion  OutDataTypeStr=double
  For Iterator : ForIterator  ResetStates=reset  IterationLimit=detectableObjects
  Selector : Selector  InputPortWidth=detectableObjects  IndexOptions=Index vector (port)  Indices=[1 3]  OutputSizes=1
  Signal2ArrayOfSignals1 : Subsystem  mask{VECTORLENGTH=detectableObjects}
      mask prompts: VECTORLENGTH "Length of vector"
  Signal2ArrayOfSignals2 : Subsystem  mask{VECTORLENGTH=detectableObjects}
      mask prompts: VECTORLENGTH "Length of vector"
  Signal2ArrayOfSignals3 : Subsystem  mask{VECTORLENGTH=detectableObjects}
      mask prompts: VECTORLENGTH "Length of vector"
  Signal2ArrayOfSignals4 : Subsystem  mask{VECTORLENGTH=detectableObjects}
      mask prompts: VECTORLENGTH "Length of vector"
  Signal2ArrayOfSignals5 : Subsystem  mask{VECTORLENGTH=detectableObjects}
      mask prompts: VECTORLENGTH "Length of vector"
  Signal2ArrayOfSignals6 : Subsystem  mask{VECTORLENGTH=detectableObjects}
      mask prompts: VECTORLENGTH "Length of vector"
  Range [m] : Outport
  Azimuth [deg] : Outport  Port=2
  Elevation [deg] : Outport  Port=3
  ID [-] : Outport  Port=4
  Velocity [m/s] : Outport  Port=5
  Heading [deg] : Outport  Port=6
  wires:
  Data Type Conversion/1 -> ID [-]/1
  Bus Selector/6 -> Signal2ArrayOfSignals6/2(Element)  "<Heading>"
  Signal2ArrayOfSignals6/1(ArrayofElements) -> Activate_Switch/2(Heading)
  Bus Selector/5 -> Signal2ArrayOfSignals5/2(Element)  "<Velocity>"
  Signal2ArrayOfSignals5/1(ArrayofElements) -> Velocity [m/s]/1
  Bus Selector/4 -> Signal2ArrayOfSignals4/2(Element)  "<ID>"
  Signal2ArrayOfSignals4/1(ArrayofElements) -> Data Type Conversion/1, Activate_Switch/1(BeamID)
  Bus Selector/3 -> Signal2ArrayOfSignals3/2(Element)  "<Elevation>"
  Signal2ArrayOfSignals3/1(ArrayofElements) -> Elevation [deg]/1
  Bus Selector/2 -> Signal2ArrayOfSignals1/2(Element)  "<Azimuth>"
  Signal2ArrayOfSignals1/1(ArrayofElements) -> Azimuth [deg]/1
  Signal2ArrayOfSignals2/1(ArrayofElements) -> Range [m]/1
  Bus Selector/1 -> Signal2ArrayOfSignals2/2(Element)  "<Range>"
  Selector/1 -> Bus Selector/1
  For Iterator/1 -> Signal2ArrayOfSignals6/1(LoopIndex), Signal2ArrayOfSignals5/1(LoopIndex), Signal2ArrayOfSignals4/1(LoopIndex), Signal2ArrayOfSignals3/1(LoopIndex), Signal2ArrayOfSignals1/1(LoopIndex), Signal2ArrayOfSignals2/1(LoopIndex), Selector/2
  AIR/1 -> Selector/1
  Activate_Switch/1(On/Off) -> Heading [deg]/1

== system Demo_TrafficFlow_cs/Citroen_C3_1/RFOBU_3_Rx_Demux ==
  Beacon_Rx : Inport
  Demux1 : Demux  Outputs=3
  Demux2 : Demux  Outputs=20
  Mux : Mux  Inputs=3
  Reorder : Subsystem  mask(Output reorder block){NrOfOutputs=20, NrOfObjects=2}
      mask prompts: NrOfOutputs "Number Of Outputs [-]"; NrOfObjects "Number Of Objects [-]"
      mask help: Change output order from object based to output type based
      mask init: same as Demo_TrafficFlow_cs/Audi_A8_1/RFOBU_1_Rx_Demux/Reorder
  xyz2GPS : PreScanUsefulBlocks/xyz2GPS  CoordinateCount=2  GPS_base=[0.000000 0.000000 0.000]
  Beacon_ID : Outport
  GPS_pos_lat_[deg] : Outport  Port=2
  GPS_pos_long_[deg] : Outport  Port=3
  GPS_pos_alt_[m] : Outport  Port=4
  BeaconType [-] : Outport  Port=5
  spare_1 : Outport  Port=6
  spare_2 : Outport  Port=7
  spare_3 : Outport  Port=8
  spare_4 : Outport  Port=9
  spare_5 : Outport  Port=10
  spare_6 : Outport  Port=11
  spare_7 : Outport  Port=12
  spare_8 : Outport  Port=13
  spare_9 : Outport  Port=14
  spare_10 : Outport  Port=15
  spare_11 : Outport  Port=16
  spare_12 : Outport  Port=17
  spare_13 : Outport  Port=18
  spare_14 : Outport  Port=19
  spare_15 : Outport  Port=20
  wires:
  Demux2/5 -> BeaconType [-]/1  "pos_z"
  Mux/1 -> xyz2GPS/1
  Demux2/20 -> spare_15/1
  Demux2/19 -> spare_14/1
  Demux2/18 -> spare_13/1
  Demux2/17 -> spare_12/1
  Demux2/16 -> spare_11/1
  Beacon_Rx/1 -> Reorder/1(In)
  Demux2/15 -> spare_10/1
  Demux2/14 -> spare_9/1
  Demux2/13 -> spare_8/1
  Demux2/12 -> spare_7/1
  Demux2/11 -> spare_6/1
  Demux2/10 -> spare_5/1
  Demux2/9 -> spare_4/1
  Demux2/8 -> spare_3/1
  Demux2/7 -> spare_2/1
  Demux2/6 -> spare_1/1  "pos_z"
  Reorder/1(Out) -> Demux2/1
  Demux1/3 -> GPS_pos_alt_[m]/1
  Demux1/2 -> GPS_pos_long_[deg]/1
  Demux1/1 -> GPS_pos_lat_[deg]/1
  xyz2GPS/1 -> Demux1/1
  Demux2/4 -> Mux/3  "pos_z"
  Demux2/3 -> Mux/2  "pos_y"
  Demux2/2 -> Mux/1  "pos_x"
  Demux2/1 -> Beacon_ID/1

== system Demo_TrafficFlow_cs/Citroen_C3_1/RFOBU_3_Tx_Mux ==
  Vehicle_Type : Inport
  spare_1 : Inport  Port=2
  spare_2 : Inport  Port=3
  spare_3 : Inport  Port=4
  spare_4 : Inport  Port=5
  spare_5 : Inport  Port=6
  spare_6 : Inport  Port=7
  spare_7 : Inport  Port=8
  spare_8 : Inport  Port=9
  spare_9 : Inport  Port=10
  spare_10 : Inport  Port=11
  spare_11 : Inport  Port=12
  spare_12 : Inport  Port=13
  spare_13 : Inport  Port=14
  spare_14 : Inport  Port=15
  spare_15 : Inport  Port=16
  Mux1 : Mux  Inputs=16
  BeaconTx : Outport
  wires:
  Vehicle_Type/1 -> Mux1/1
  Mux1/1 -> BeaconTx/1
  spare_10/1 -> Mux1/11
  spare_9/1 -> Mux1/10
  spare_8/1 -> Mux1/9
  spare_7/1 -> Mux1/8
  spare_6/1 -> Mux1/7
  spare_5/1 -> Mux1/6
  spare_4/1 -> Mux1/5
  spare_3/1 -> Mux1/4
  spare_2/1 -> Mux1/3
  spare_1/1 -> Mux1/2
  spare_15/1 -> Mux1/16
  spare_14/1 -> Mux1/15
  spare_13/1 -> Mux1/14
  spare_12/1 -> Mux1/13
  spare_11/1 -> Mux1/12

== system Demo_TrafficFlow_cs/Citroen_C3_1/SELF_Demux ==
  SELF : Inport
  Demux1 : Demux  Outputs=3
  Demux2 : Demux  Outputs=3
  Demux3 : Demux  Outputs=[3 3 1 1]
  Demux4 : Demux  Outputs=3
  Gain : Gain  Gain=180/pi
  Gain1 : Gain  Gain=180/pi
  Selector : Selector  InputPortWidth=3  IndexOptions=Index vector (dialog)  Indices=[3]  OutputSizes=1
  compas converter : Subsystem
  xyz2GPS : PreScanUsefulBlocks/xyz2GPS  CoordinateCount=1  GPS_base=[0.000000 0.000000 0.000]
  x [m] : Outport
  y [m] : Outport  Port=2
  z [m] : Outport  Port=3
  rotx [deg] : Outport  Port=4
  roty [deg] : Outport  Port=5
  rotz [deg] : Outport  Port=6
  GPS lat [deg] : Outport  Port=7
  GPS long [deg] : Outport  Port=8
  GPS alt [m] : Outport  Port=9
  velocity [m/s] : Outport  Port=10
  heading [deg] : Outport  Port=11
  yaw rate [deg/s] : Outport  Port=12
  wires:
  Demux3/4 -> Gain1/1
  Gain1/1 -> yaw rate [deg/s]/1
  Demux3/3 -> velocity [m/s]/1
  compas converter/1(heading [deg]) -> heading [deg]/1
  xyz2GPS/1 -> Demux4/1
  Demux4/3 -> GPS alt [m]/1
  Demux4/2 -> GPS long [deg]/1
  Demux4/1 -> GPS lat [deg]/1
  Demux3/2 -> Gain/1, Selector/1
  Demux3/1 -> xyz2GPS/1, Demux1/1
  SELF/1 -> Demux3/1
  Demux2/3 -> rotz [deg]/1
  Demux2/2 -> roty [deg]/1
  Demux2/1 -> rotx [deg]/1
  Demux1/3 -> z [m]/1
  Demux1/2 -> y [m]/1
  Demux1/1 -> x [m]/1
  Gain/1 -> Demux2/1
  Selector/1 -> compas converter/1(rotz [rad])

== system Demo_TrafficFlow_cs/Citroen_C3_1/Simple_Longitudinal_ACC ==
  note: v_ini=50 km/h
  Advised Velocity [km/h] : Inport
  Range [m] : Inport  Port=2
  Velocity [m/s] : Inport  Port=3
  HOLD Advised Speed : Subsystem
  HardCoded Safety Distance [m] : Constant  Value=15
  LONGITUDINAL ACC & Dynamics : Subsystem
  Manual Switch : simulink/Signal Routing/Manual Switch  SystemSampleTime=-1  sw=1  action=0  varsize=off
  Manual Switch1 : simulink/Signal Routing/Manual Switch  SystemSampleTime=-1  sw=0  action=0  varsize=off
  Memory2 : Memory
  Own Speed Intention [km/h] : Constant  Value=60
  Product1 : Product  RndMeth=Zero
  TimeToCollision [s] Velocity Dependent Safety Distance : Constant
  ACC_Velocity [m/s] : Outport
  wires:
  Range [m]/1 -> LONGITUDINAL ACC & Dynamics/1(Detected Range [m])
  TimeToCollision [s] Velocity Dependent Safety Distance/1 -> Product1/1
  HardCoded Safety Distance [m]/1 -> Manual Switch/1
  Product1/1 -> Manual Switch/2
  Manual Switch/1 -> LONGITUDINAL ACC & Dynamics/4(Safety Distance [m])
  Advised Velocity [km/h]/1 -> HOLD Advised Speed/1(v)
  Own Speed Intention [km/h]/1 -> Manual Switch1/2
  HOLD Advised Speed/1(v_hold) -> Manual Switch1/1
  Manual Switch1/1 -> LONGITUDINAL ACC & Dynamics/3(Own Velocity Intention [km/h])
  LONGITUDINAL ACC & Dynamics/1(Velocity [m/s]) -> ACC_Velocity [m/s]/1, Memory2/1
  Velocity [m/s]/1 -> LONGITUDINAL ACC & Dynamics/2(Detected Velocity Vehicle [m/s])
  Memory2/1 -> Product1/2
